simulink model "BswSigProc"  (R2022b)
settings: Solver=FixedStepDiscrete  StartTime=0.0  StopTime=10.0  FixedStep=0.01  RelTol=1e-3
model workspace (MAT data in the model file):
  NvMBlockRecordSignal = Simulink.Signal
  NvMBlockRecordSignal.DataType = 'UInt8'
  NvMBlockRecordSignal.Dimensions = [16 1]
  NvMBlockRecordSignal.DimensionsMode = 'auto'
  NvMBlockRecordSignal.Complexity = 'real'
  NvMBlockRecordSignal.SampleTime = -1
  NvMBlockRecordSignal.SamplingMode = 'auto'
  NvMBlockRecordSignal.InitialValue = 'NvMBlockRecordSignalInit'
  NvMBlockRecordSignalInit = Simulink.Parameter
  NvMBlockRecordSignalInit.Value = [255; 255; 255; 255; 255; 255; 255; 255; 255; 255; 255; 255; 255; 255; 255; 255]
  NvMBlockRecordSignalInit.DataType = 'UInt8'
  NvMBlockRecordSignalInit.Complexity = 'real'
  NvMBlockRecordSignalInit.Dimensions = [16 1]
(part 1 of 2)
settings Model: Creator=blue.ge  LastModifiedBy=blue.ge
settings DataIOCC: SaveFormat=StructureWithTime
settings OptimizationCC: DefaultParameterBehavior=Inlined  EnhancedBackFolding=on  PassReuseOutputArgsAs=Individual arguments  ZeroExternalMemoryAtStartup=off  ZeroInternalMemoryAtStartup=off  OptimizeBlockOrder=speed  DifferentSizesBufferReuse=on  OptimizationCustomize=off  EfficientTunableParamExpr=on
settings DebuggingCC: ParameterTunabilityLossMsg=error
settings ModelReferenceCC: ModelReferenceNumInstancesAllowed=Single
settings SFSimCC: MATLABDynamicMemAlloc=off
settings RTWCC: Description=AUTOSAR  SystemTargetFile=autosar.tlc  GenCodeOnly=on  TemplateMakefile=ert_default_tmf
settings CodeAppCC: ForceParamTrailComments=on  IgnoreCustomStorageClasses=off  OperatorAnnotations=on  SimulinkDataObjDesc=on  SFDataObjDesc=on  InsertBlockDesc=on
settings STFCustomTargetCC: TargetLangStandard=C99 (ISO)  MultiwordLength=256  ParMdlRefBuildCompliant=on  CompOptLevelCompliant=on  IncludeMdlTerminateFcn=off  CombineOutputUpdateFcns=on  SuppressErrorStatus=on  ERTCustomFileBanners=on  SupportNonFinite=off  SupportComplex=off  SupportContinuousTime=off  SupportNonInlinedSFcns=off  RemoveResetFunc=on  MATLABClassNameForMDSCustomization=Simulink.SoftwareTarget.ERTCustomization  AutosarCompliant=on  UseToolchainInfoCompliant=on  LookupTableObjectStructAxisOrder=2,1,3,4,...  LUTObjectStructOrderEvenSpacing=Size,Table,Breakpoints  SystemTargetFile=autosar.tlc
settings ERTTargetCC: TargetLangStandard=C99 (ISO)  ConcurrentExecutionCompliant=off  IncludeMdlTerminateFcn=off  SuppressErrorStatus=on  SupportNonFinite=off  SupportComplex=off  ModelStepFunctionPrototypeControlCompliant=off  CPPClassGenCompliant=off  AutosarCompliant=on  LookupTableObjectStructAxisOrder=2,1,3,4,...  LUTObjectStructOrderEvenSpacing=Size,Table,Breakpoints  InlinedParameterPlacement=NonHierarchical  ERTCustomFileTemplate=example_file_process.tlc

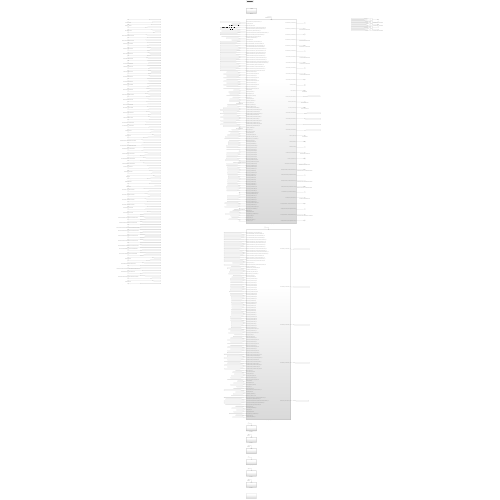
[thumbnail: Simulink's stored preview of the top-level diagram]
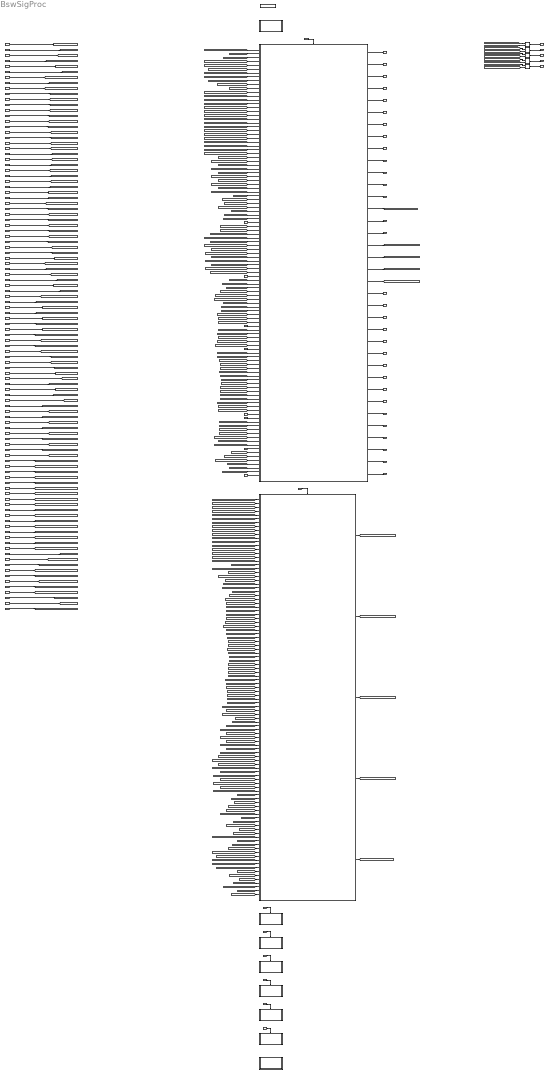
== system BswSigProc ==
  BswSigProc_Runnable : Inport  SampleTime=0.02  OutputFunctionCall=on
  BswSigProc_SignalDeal : Inport  Port=2  SampleTime=0.05  OutputFunctionCall=on
  Xcp_Event0 : Inport  Port=3  SampleTime=0.01  OutputFunctionCall=on
  Xcp_Event1 : Inport  Port=4  SampleTime=0.02  OutputFunctionCall=on
  Xcp_Event2 : Inport  Port=5  SampleTime=0.05  OutputFunctionCall=on
  Xcp_Event3 : Inport  Port=6  SampleTime=0.1  OutputFunctionCall=on
  Xcp_Event4 : Inport  Port=7  SampleTime=0.2  OutputFunctionCall=on
  Xcp_Event5 : Inport  Port=8  SampleTime=1  OutputFunctionCall=on
  ClimaTiMgrVFCInfotainmentPush_ClimaTiMgrVFCInfotainmentPush : Inport  Port=9  OutDataTypeStr=Enum: OnOff1  PortDimensions=1
  ClimRlyCmd_ClimRlyCmd : Inport  Port=10  OutDataTypeStr=Enum: OnOff1  PortDimensions=1
  CmptmtTFrntRaw_CmptmtTFrntRaw : Inport  Port=11  OutDataTypeStr=Bus: CmptmtTFrnt  PortDimensions=1  BusOutputAsStruct=on
  PrkgClimaActvMgrVFCBodyPreClimatisation_PrkgClimaActvMgrVFCBodyPreClimatisation : Inport  Port=12  OutDataTypeStr=Boolean  PortDimensions=1
  PrkgClimaActvMgrVFCGlobalShortDuration_PrkgClimaActvMgrVFCGlobalShortDuration : Inport  Port=13  OutDataTypeStr=Boolean  PortDimensions=1
  PrkgClimaActvMgrVFCLocking_PrkgClimaActvMgrVFCLocking : Inport  Port=14  OutDataTypeStr=Boolean  PortDimensions=1
  PrkgClimaActvMgrVFCParkingDrivingClimatization_PrkgClimaActvMgrVFCParkingDrivingClimatization : Inport  Port=15  OutDataTypeStr=Boolean  PortDimensions=1
  PrkgClimaActvMgrVFCPostClimatisation_PrkgClimaActvMgrVFCPostClimatisation : Inport  Port=16  OutDataTypeStr=Boolean  PortDimensions=1
  PrkgClimaActvMgrVFCWindows_PrkgClimaActvMgrVFCWindows : Inport  Port=17  OutDataTypeStr=Boolean  PortDimensions=1
  PwrGernalOn2OffCnt_PwrMdGernalOn2OffCnt : Inport  Port=18  OutDataTypeStr=On2OffCnt  PortDimensions=1
  RlyCmdCmft_RlyCmdCmft : Inport  Port=19  OutDataTypeStr=Enum: OnOff1  PortDimensions=1
  ActrPosnFrntActForOsaAndRecirc_ActrPosnFrntActForOsaAndRecirc : Inport  Port=20  OutMin=0  OutMax=100  OutDataTypeStr=Perc9  PortDimensions=1
  ActrPosnFrntActForRowFirstModDistbn_ActrPosnFrntActForRowFirstModDistbn : Inport  Port=21  OutMin=0  OutMax=100  OutDataTypeStr=Perc9  PortDimensions=1
  ActrPosnFrntActForRowSecModDistbnLe_ActrPosnFrntActForRowSecModDistbnLe : Inport  Port=22  OutMin=0  OutMax=100  OutDataTypeStr=Perc9  PortDimensions=1
  ActrPosnFrntActForRowSecModDistbnRi_ActrPosnFrntActForRowSecModDistbnRi : Inport  Port=23  OutMin=0  OutMax=100  OutDataTypeStr=Perc9  PortDimensions=1
  AirFlapPosnFrntActForRowFirstForActrDefrst_AirFlapPosnFrntActForRowFirstForActrDefrst : Inport  Port=24  OutMin=0  OutMax=100  OutDataTypeStr=Perc9  PortDimensions=1
  AirFlapPosnFrntActForRowFirstForActrFlrLe_AirFlapPosnFrntActForRowFirstForActrFlrLe : Inport  Port=25  OutMin=0  OutMax=100  OutDataTypeStr=Perc9  PortDimensions=1
  AirFlapPosnFrntActForRowFirstForActrFlrRi_AirFlapPosnFrntActForRowFirstForActrFlrRi : Inport  Port=26  OutMin=0  OutMax=100  OutDataTypeStr=Perc9  PortDimensions=1
  AirFlapPosnFrntActForRowFirstForActrTLe_AirFlapPosnFrntActForRowFirstForActrTLe : Inport  Port=27  OutMin=0  OutMax=100  OutDataTypeStr=Perc9  PortDimensions=1
  AirFlapPosnFrntActForRowFirstForActrTRi_AirFlapPosnFrntActForRowFirstForActrTRi : Inport  Port=28  OutMin=0  OutMax=100  OutDataTypeStr=Perc9  PortDimensions=1
  AirFlapPosnFrntActForRowFirstForActrVentLe_AirFlapPosnFrntActForRowFirstForActrVentLe : Inport  Port=29  OutMin=0  OutMax=100  OutDataTypeStr=Perc9  PortDimensions=1
  AirFlapPosnFrntActForRowFirstForActrVentRi_AirFlapPosnFrntActForRowFirstForActrVentRi : Inport  Port=30  OutMin=0  OutMax=100  OutDataTypeStr=Perc9  PortDimensions=1
  AirFlapPosnFrntActForRowFirstForActrVentSideLe_AirFlapPosnFrntActForRowFirstForActrVentSideLe : Inport  Port=31  OutMin=0  OutMax=100  OutDataTypeStr=Perc9  PortDimensions=1
  AirFlapPosnFrntActForRowFirstForActrVentSideRi_AirFlapPosnFrntActForRowFirstForActrVentSideRi : Inport  Port=32  OutMin=0  OutMax=100  OutDataTypeStr=Perc9  PortDimensions=1
  AirFlapPosnFrntActForRowFirstForOsa_AirFlapPosnFrntActForRowFirstForOsa : Inport  Port=33  OutMin=0  OutMax=100  OutDataTypeStr=Perc9  PortDimensions=1
  AirFlapPosnFrntActForRowFirstForRecirc_AirFlapPosnFrntActForRowFirstForRecirc : Inport  Port=34  OutMin=0  OutMax=100  OutDataTypeStr=Perc9  PortDimensions=1
  AirFlapPosnFrntActForRowSecForActrTLe_AirFlapPosnFrntActForRowSecForActrTLe : Inport  Port=35  OutMin=0  OutMax=100  OutDataTypeStr=Perc9  PortDimensions=1
  AirFlapPosnFrntActForRowSecForActrTRi_AirFlapPosnFrntActForRowSecForActrTRi : Inport  Port=36  OutMin=0  OutMax=100  OutDataTypeStr=Perc9  PortDimensions=1
  AirFragCh1RunngSts_AirFragCh1RunngSts : Inport  Port=37  OutDataTypeStr=Enum: OnOff1  PortDimensions=1
  AirFragCh1RunngSts_AirFragCh1RunngSts_IsUpdated : Inport  Port=38  OutDataTypeStr=boolean  PortDimensions=1
  AirFragCh2RunngSts_AirFragCh2RunngSts : Inport  Port=39  OutDataTypeStr=Enum: OnOff1  PortDimensions=1
  AirFragCh2RunngSts_AirFragCh2RunngSts_IsUpdated : Inport  Port=40  OutDataTypeStr=boolean  PortDimensions=1
  AirFragCh3RunngSts_AirFragCh3RunngSts : Inport  Port=41  OutDataTypeStr=Enum: OnOff1  PortDimensions=1
  AirFragCh3RunngSts_AirFragCh3RunngSts_IsUpdated : Inport  Port=42  OutDataTypeStr=boolean  PortDimensions=1
  AirFragCh4RunngSts_AirFragCh4RunngSts : Inport  Port=43  OutDataTypeStr=Enum: OnOff1  PortDimensions=1
  AirFragCh4RunngSts_AirFragCh4RunngSts_IsUpdated : Inport  Port=44  OutDataTypeStr=boolean  PortDimensions=1
  AirFragCh5RunngSts_AirFragCh5RunngSts : Inport  Port=45  OutDataTypeStr=Enum: OnOff1  PortDimensions=1
  AirFragCh5RunngSts_AirFragCh5RunngSts_IsUpdated : Inport  Port=46  OutDataTypeStr=boolean  PortDimensions=1
  AmbTRaw_AmbTRaw : Inport  Port=47  OutDataTypeStr=Bus: AmbTWithQly  PortDimensions=1  BusOutputAsStruct=on
  BatteryVolValue_BatteryVolValue : Inport  Port=48  OutDataTypeStr=BatteryVolValue  PortDimensions=1
  BkpOfDstTrvld_BkpOfDstTrvld : Inport  Port=49  OutDataTypeStr=Len31  PortDimensions=1
  BkpOfDstTrvld_BkpOfDstTrvld_IsUpdated : Inport  Port=50  OutDataTypeStr=boolean  PortDimensions=1
  CarTiGlb_CarTiGlb : Inport  Port=51  OutDataTypeStr=CarTiGlb  PortDimensions=1
  CarTiGlb_CarTiGlb_IsUpdated : Inport  Port=52  OutDataTypeStr=boolean  PortDimensions=1
  CmptmtTRearRaw_CmptmtTRearRaw : Inport  Port=53  OutDataTypeStr=Bus: CmptmtTFrnt  PortDimensions=1  BusOutputAsStruct=on
  EvaprTReRaw_EvaprTReRaw : Inport  Port=54  OutDataTypeStr=Bus: EvaprTFrnt  PortDimensions=1  BusOutputAsStruct=on
  FrntHvacBlowerSts_FrntHvacBlowerSts : Inport  Port=55  OutDataTypeStr=Enum: HvacFanSts  PortDimensions=1
  HmiHvacFanLvlFrnt_HmiHvacFanLvlFrnt : Inport  Port=56  OutDataTypeStr=Enum: HmiHvacFanLvl  PortDimensions=1
  HvacFanSts2HvacFanBattI2_HvacFanSts2HvacFanBattI2 : Inport  Port=57  OutMin=0  OutMax=63.25  OutDataTypeStr=BlowerReI  PortDimensions=1
  HvacFanSts2HvacFanBattI2_HvacFanSts2HvacFanBattI2_IsUpdated : Inport  Port=58  OutDataTypeStr=boolean  PortDimensions=1
  HvacFanSts2HvacFanBattU2_HvacFanSts2HvacFanBattU2 : Inport  Port=59  OutMin=0  OutMax=25.400000000000002  OutDataTypeStr=U8  PortDimensions=1
  HvacFanSts2HvacFanBattU2_HvacFanSts2HvacFanBattU2_IsUpdated : Inport  Port=60  OutDataTypeStr=boolean  PortDimensions=1
  HvacFanSts3HvacFanBattI_HvacFanSts3HvacFanBattI : Inport  Port=61  OutDataTypeStr=BlowerBattI  PortDimensions=1
  HvacFanSts3HvacFanBattI_HvacFanSts3HvacFanBattI_IsUpdated : Inport  Port=62  OutDataTypeStr=boolean  PortDimensions=1
  HvacFanSts3HvacFanBattU_HvacFanSts3HvacFanBattU : Inport  Port=63  OutDataTypeStr=U10  PortDimensions=1
  HvacFanSts3HvacFanBattU_HvacFanSts3HvacFanBattU_IsUpdated : Inport  Port=64  OutDataTypeStr=boolean  PortDimensions=1
  HvacFanSts3HvacFanSpdFd_HvacFanSts3HvacFanSpdFd : Inport  Port=65  OutDataTypeStr=BlowerSpd  PortDimensions=1
  HvacFanSts3HvacFanSpdFd_HvacFanSts3HvacFanSpdFd_IsUpdated : Inport  Port=66  OutDataTypeStr=boolean  PortDimensions=1
  HvacOsaAndRecActrQfForHp_HvacOsaAndRecActrQfForHp : Inport  Port=67  OutDataTypeStr=Enum: Err1  PortDimensions=1
  HvacReHexAirT_HvacReHexAirT : Inport  Port=68  OutDataTypeStr=Bus: HvacAirTForHeatrFrnt  PortDimensions=1  BusOutputAsStruct=on
  IntCo2Conc_IntCo2Conc : Inport  Port=69  OutDataTypeStr=Co2Conc  PortDimensions=1
  IntCo2Conc_IntCo2Conc_IsUpdated : Inport  Port=70  OutDataTypeStr=boolean  PortDimensions=1
  IntCo2RunSts_IntCo2RunSts : Inport  Port=71  OutDataTypeStr=Enum: PmSnsrSts  PortDimensions=1
  IntCo2RunSts_IntCo2RunSts_IsUpdated : Inport  Port=72  OutDataTypeStr=boolean  PortDimensions=1
  RlyCmftForClimaReq_Ngcc_RlyCmftForClimaReq : Inport  Port=73  OutDataTypeStr=Enum: OnOff1  PortDimensions=1
  RlyCrashForClimaReq_Ngcc_RlyCrashForClimaReq : Inport  Port=74  OutDataTypeStr=Enum: OnOff1  PortDimensions=1
  SysServCarCfgSts_SysServCarID : Inport  Port=75  OutDataTypeStr=Enum: SysServCarID  PortDimensions=1
  SysServCarCfgSts_SysServCCPAQSSts : Inport  Port=76  OutDataTypeStr=UInt8  PortDimensions=1
  SysServCarCfgSts_SysServCCPBGMNum : Inport  Port=77  OutDataTypeStr=UInt8  PortDimensions=1
  SysServCarCfgSts_SysServCCPHEXSensorSts : Inport  Port=78  OutDataTypeStr=UInt8  PortDimensions=1
  SysServCarCfgSts_SysServCCPLin2AFUSts : Inport  Port=79  OutDataTypeStr=Boolean  PortDimensions=1
  SysServCarCfgSts_SysServCCPLin2AIUSts : Inport  Port=80  OutDataTypeStr=UInt8  PortDimensions=1
  SysServCarCfgSts_SysServCCPLin2AQSSts : Inport  Port=81  OutDataTypeStr=Boolean  PortDimensions=1
  SysServCarCfgSts_SysServCCPLin2CDSSts : Inport  Port=82  OutDataTypeStr=Boolean  PortDimensions=1
  SysServCarCfgSts_SysServCCPLin2PMSISts : Inport  Port=83  OutDataTypeStr=Boolean  PortDimensions=1
  SysServCarCfgSts_SysServCCPLin3VGASts : Inport  Port=84  OutDataTypeStr=Boolean  PortDimensions=1
  SysServCarCfgSts_SysServDUAirVentSnrSts : Inport  Port=85  OutDataTypeStr=Boolean  PortDimensions=1
  SysServCarCfgSts_SysServDUBlowerPwmSignSts : Inport  Port=86  OutDataTypeStr=Boolean  PortDimensions=1
  SysServCarCfgSts_SysServDUBlowerPwmSignTypeSts : Inport  Port=87  OutDataTypeStr=UInt8  PortDimensions=1
  SysServCarCfgSts_SysServDUBlowerPwmSts : Inport  Port=88  OutDataTypeStr=Boolean  PortDimensions=1
  SysServCarCfgSts_SysServDUDCDefrostSts : Inport  Port=89  OutDataTypeStr=UInt8  PortDimensions=1
  SysServCarCfgSts_SysServDUDCFLMdeSts : Inport  Port=90  OutDataTypeStr=UInt8  PortDimensions=1
  SysServCarCfgSts_SysServDUDCModeSts : Inport  Port=91  OutDataTypeStr=UInt8  PortDimensions=1
  SysServCarCfgSts_SysServDUDCRecSts : Inport  Port=92  OutDataTypeStr=UInt8  PortDimensions=1
  SysServCarCfgSts_SysServDUDCSModeSts : Inport  Port=93  OutDataTypeStr=UInt8  PortDimensions=1
  SysServCarCfgSts_SysServDUDCSTLSts : Inport  Port=94  OutDataTypeStr=UInt8  PortDimensions=1
  SysServCarCfgSts_SysServDUDCTLSts : Inport  Port=95  OutDataTypeStr=UInt8  PortDimensions=1
  SysServCarCfgSts_SysServDUDCTRSts : Inport  Port=96  OutDataTypeStr=UInt8  PortDimensions=1
  SysServCarCfgSts_SysServDUFLFootSts : Inport  Port=97  OutDataTypeStr=UInt8  PortDimensions=1
  SysServCarCfgSts_SysServDUFLVentSts : Inport  Port=98  OutDataTypeStr=UInt8  PortDimensions=1
  SysServCarCfgSts_SysServDUFRFootSts : Inport  Port=99  OutDataTypeStr=UInt8  PortDimensions=1
  SysServCarCfgSts_SysServDUFRVentSts : Inport  Port=100  OutDataTypeStr=UInt8  PortDimensions=1
  SysServCarCfgSts_SysServDUIncarMotorSts : Inport  Port=101  OutDataTypeStr=Boolean  PortDimensions=1
  SysServCarCfgSts_SysServDULin1HBMFSts : Inport  Port=102  OutDataTypeStr=Boolean  PortDimensions=1
  SysServCarCfgSts_SysServDULin4HFAFSts : Inport  Port=103  OutDataTypeStr=UInt32  PortDimensions=1
  SysServCarCfgSts_SysServDUReBlowerPwmSignSts : Inport  Port=104  OutDataTypeStr=UInt8  PortDimensions=1
  SysServCarCfgSts_SysServDUReBlowerPwmSts : Inport  Port=105  OutDataTypeStr=UInt8  PortDimensions=1
  SysServCarCfgSts_SysServDUSFLFootSts : Inport  Port=106  OutDataTypeStr=UInt8  PortDimensions=1
  SysServCarCfgSts_SysServDUSFLVentSts : Inport  Port=107  OutDataTypeStr=UInt8  PortDimensions=1
  SysServCarCfgSts_SysServDUSFRFootSts : Inport  Port=108  OutDataTypeStr=UInt8  PortDimensions=1
  SysServCarCfgSts_SysServDUSFRVentSts : Inport  Port=109  OutDataTypeStr=UInt8  PortDimensions=1
  SysServCarCfgSts_SysServDUSnsrForFCmptmtTSts : Inport  Port=110  OutDataTypeStr=UInt8  PortDimensions=1
  SysServCarCfgSts_SysServDUSnsrForRair : Inport  Port=111  OutDataTypeStr=UInt8  PortDimensions=1
  SysServCarCfgSts_SysServDUSnsrForRCmptmtTSts : Inport  Port=112  OutDataTypeStr=UInt8  PortDimensions=1
  SysServCarCfgSts_SysServLowSideRETXVSts : Inport  Port=113  OutDataTypeStr=UInt8  PortDimensions=1
  VehBattU_VehBattU : Inport  Port=114  OutDataTypeStr=Bus: SysU  PortDimensions=1  BusOutputAsStruct=on
  VehBattU_VehBattU_IsUpdated : Inport  Port=115  OutDataTypeStr=boolean  PortDimensions=1
  VehModMngtGlbSafe1_Ngcc_VehModMngtGlbSafe1 : Inport  Port=116  OutDataTypeStr=Bus: VehModMngtGlbSafe1  PortDimensions=1  BusOutputAsStruct=on
  VehSpdLgt_Ngcc_VehSpdLgt : Inport  Port=117  OutDataTypeStr=Bus: VehSpdLgtSafe1  PortDimensions=1  BusOutputAsStruct=on
  VFCInfoEna_VFCInfoEna : Inport  Port=118  OutDataTypeStr=Enum: EnableDisableCoding  PortDimensions=1
  VFCInfoEna_VFCInfoEna_IsUpdated : Inport  Port=119  OutDataTypeStr=boolean  PortDimensions=1
  BswLifeCycle_LifeCycle : Inport  Port=120  OutDataTypeStr=Enum: CCMLifeCycleModeGroup  PortDimensions=1
  BswSigProcDtcService : Subsystem
  BswSigProc_Init : Subsystem
  BswSigProc_Runnable_sys : Subsystem
  BswSigProc_SignalDeal_sys : Subsystem
  CcmIntelliClimaResd1_CcmIntelliClimaResd1_merge : Merge
  CcmIntelliClimaResd2_CcmIntelliClimaResd2_merge : Merge
  CcmIntelliClimaResd3_CcmIntelliClimaResd3_merge : Merge
  CcmIntelliClimaResd4_CcmIntelliClimaResd4_merge : Merge
  HvacRecFlapActlPosn_HvacRecFlapActlPosn_merge : Merge
  NvMBlockRecordSignal : DataStoreMemory  DataStoreName=NvMBlockRecordSignal  InitialValue=NvMBlockRecordSignalInit  ShowAdditionalParam=off  Dimensions=16  StateMustResolveToSignalObject=on
  Xcp_Event0_sys : Subsystem
  Xcp_Event1_sys : Subsystem
  Xcp_Event2_sys : Subsystem
  Xcp_Event3_sys : Subsystem
  Xcp_Event4_sys : Subsystem
  Xcp_Event5_sys : Subsystem
  CcmIntelliClimaResd10_CcmIntelliClimaResd10 : Outport  OutDataTypeStr=UInt16  PortDimensions=1
  CcmIntelliClimaResd11_CcmIntelliClimaResd11 : Outport  Port=2  OutDataTypeStr=UInt16  PortDimensions=1
  CcmIntelliClimaResd12_CcmIntelliClimaResd12 : Outport  Port=3  OutDataTypeStr=UInt16  PortDimensions=1
  CcmIntelliClimaResd13_CcmIntelliClimaResd13 : Outport  Port=4  OutDataTypeStr=UInt16  PortDimensions=1
  CcmIntelliClimaResd14_CcmIntelliClimaResd14 : Outport  Port=5  OutDataTypeStr=UInt16  PortDimensions=1
  CcmIntelliClimaResd15_CcmIntelliClimaResd15 : Outport  Port=6  OutDataTypeStr=UInt16  PortDimensions=1
  CcmIntelliClimaResd5_CcmIntelliClimaResd5 : Outport  Port=7  OutDataTypeStr=UInt16  PortDimensions=1
  CcmIntelliClimaResd6_CcmIntelliClimaResd6 : Outport  Port=8  OutDataTypeStr=UInt16  PortDimensions=1
  CcmIntelliClimaResd7_CcmIntelliClimaResd7 : Outport  Port=9  OutDataTypeStr=UInt16  PortDimensions=1
  CcmIntelliClimaResd8_CcmIntelliClimaResd8 : Outport  Port=10  OutDataTypeStr=UInt16  PortDimensions=1
  CcmIntelliClimaResd9_CcmIntelliClimaResd9 : Outport  Port=11  OutDataTypeStr=UInt16  PortDimensions=1
  CmptmtTRe_CmptmtTRe : Outport  Port=12  OutDataTypeStr=Bus: CmptmtTFrnt  PortDimensions=1  BusOutputAsStruct=on
  EvaprTRe_EvaprTRe : Outport  Port=13  OutDataTypeStr=Bus: EvaprTFrnt  PortDimensions=1  BusOutputAsStruct=on
  HvacRecFlapActlPosn_HvacRecFlapActlPosn : Outport  Port=14  OutMin=0  OutMax=100  OutDataTypeStr=Perc9  PortDimensions=1
  ReHvacHexAirT_ReHvacHexAirT : Outport  Port=15  OutDataTypeStr=Bus: HvacAirTForHeatrFrnt  PortDimensions=1  BusOutputAsStruct=on
  VFCVectorCCM_VFCVectorCCM : Outport  Port=16  OutDataTypeStr=Bus: VFCVector  PortDimensions=1  BusOutputAsStruct=on
  CcmIntelliClimaResd1_CcmIntelliClimaResd1 : Outport  Port=17  OutDataTypeStr=UInt8  PortDimensions=1
  CcmIntelliClimaResd2_CcmIntelliClimaResd2 : Outport  Port=18  OutDataTypeStr=UInt8  PortDimensions=1
  CcmIntelliClimaResd3_CcmIntelliClimaResd3 : Outport  Port=19  OutDataTypeStr=UInt8  PortDimensions=1
  CcmIntelliClimaResd4_CcmIntelliClimaResd4 : Outport  Port=20  OutDataTypeStr=UInt8  PortDimensions=1
  CmptmtTFrnt_CmptmtTFrnt : Outport  Port=21  OutDataTypeStr=Bus: CmptmtTFrnt  PortDimensions=1  BusOutputAsStruct=on
  ComMPNCState_PNCState : Outport  Port=22  OutDataTypeStr=Bus: PNCState  PortDimensions=1  BusOutputAsStruct=on
  ComMPNCState_VFCState : Outport  Port=23  OutDataTypeStr=UInt16  PortDimensions=1
  FrntHvacBlowerSts_sdb_FrntHvacBlowerSts : Outport  Port=24  OutDataTypeStr=Enum: HvacFanSts  PortDimensions=1
  HvacCondsorT_sdb_HvacCondsorT : Outport  Port=25  OutDataTypeStr=Bus: EvaprTFrnt  PortDimensions=1  BusOutputAsStruct=on
  HvacDefrstFlapActlPosn_HvacDefrstFlapActlPosn : Outport  Port=26  OutMin=0  OutMax=101  OutDataTypeStr=Perc101  PortDimensions=1
  HvacModFlapActlPosnFrstRowLe_HvacModFlapActlPosnFrstRowLe : Outport  Port=27  OutMin=0  OutMax=101  OutDataTypeStr=Perc101  PortDimensions=1
  HvacModFlapActlPosnFrstRowRi_HvacModFlapActlPosnFrstRowRi : Outport  Port=28  OutMin=0  OutMax=101  OutDataTypeStr=Perc101  PortDimensions=1
  HvacModFlapActlPosnSecRowLe_HvacModFlapActlPosnSecRowLe : Outport  Port=29  OutMin=0  OutMax=101  OutDataTypeStr=Perc101  PortDimensions=1
  HvacModFlapActlPosnSecRowRi_HvacModFlapActlPosnSecRowRi : Outport  Port=30  OutMin=0  OutMax=101  OutDataTypeStr=Perc101  PortDimensions=1
  HvacOsaAndRecActrQfForHp_sdb_HvacOsaAndRecActrQfForHp : Outport  Port=31  OutDataTypeStr=Enum: Err1  PortDimensions=1
  HvacOsaFlapActlPosn_HvacOsaFlapActlPosn : Outport  Port=32  OutMin=0  OutMax=100  OutDataTypeStr=Perc9  PortDimensions=1
  HvacTempFlapActlPosnFrstRowLe_HvacTempFlapActlPosnFrstRowLe : Outport  Port=33  OutMin=0  OutMax=101  OutDataTypeStr=Perc101  PortDimensions=1
  HvacTempFlapActlPosnFrstRowRi_HvacTempFlapActlPosnFrstRowRi : Outport  Port=34  OutMin=0  OutMax=101  OutDataTypeStr=Perc101  PortDimensions=1
  HvacTempFlapActlPosnSecRowLe_HvacTempFlapActlPosnSecRowLe : Outport  Port=35  OutMin=0  OutMax=101  OutDataTypeStr=Perc101  PortDimensions=1
  HvacTempFlapActlPosnSecRowRi_HvacTempFlapActlPosnSecRowRi : Outport  Port=36  OutMin=0  OutMax=101  OutDataTypeStr=Perc101  PortDimensions=1
  wires:
  EvaprTReRaw_EvaprTReRaw/1 -> BswSigProc_Runnable_sys/46(EvaprTReRaw_EvaprTReRaw_read)
  HvacReHexAirT_HvacReHexAirT/1 -> BswSigProc_Runnable_sys/60(HvacReHexAirT_HvacReHexAirT_read)
  SysServCarCfgSts_SysServCCPLin2AQSSts/1 -> BswSigProc_Runnable_sys/73(SysServCarCfgSts_SysServCCPLin2AQSSts_read)
  SysServCarCfgSts_SysServDUBlowerPwmSignTypeSts/1 -> BswSigProc_Runnable_sys/79(SysServCarCfgSts_SysServDUBlowerPwmSignTypeSts_read)
  SysServCarCfgSts_SysServDUReBlowerPwmSignSts/1 -> BswSigProc_Runnable_sys/96(SysServCarCfgSts_SysServDUReBlowerPwmSignSts_read)
  SysServCarCfgSts_SysServDUReBlowerPwmSts/1 -> BswSigProc_Runnable_sys/97(SysServCarCfgSts_SysServDUReBlowerPwmSts_read)
  SysServCarCfgSts_SysServLowSideRETXVSts/1 -> BswSigProc_Runnable_sys/105(SysServCarCfgSts_SysServLowSideRETXVSts_read)
  BswSigProc_Runnable_sys/1(CcmIntelliClimaResd10_CcmIntelliClimaResd10_write) -> CcmIntelliClimaResd10_CcmIntelliClimaResd10/1
  BswSigProc_Runnable_sys/2(CcmIntelliClimaResd11_CcmIntelliClimaResd11_write) -> CcmIntelliClimaResd11_CcmIntelliClimaResd11/1
  BswSigProc_Runnable_sys/3(CcmIntelliClimaResd12_CcmIntelliClimaResd12_write) -> CcmIntelliClimaResd12_CcmIntelliClimaResd12/1
  BswSigProc_Runnable_sys/4(CcmIntelliClimaResd13_CcmIntelliClimaResd13_write) -> CcmIntelliClimaResd13_CcmIntelliClimaResd13/1
  BswSigProc_Runnable_sys/5(CcmIntelliClimaResd14_CcmIntelliClimaResd14_write) -> CcmIntelliClimaResd14_CcmIntelliClimaResd14/1
  BswSigProc_Runnable_sys/6(CcmIntelliClimaResd15_CcmIntelliClimaResd15_write) -> CcmIntelliClimaResd15_CcmIntelliClimaResd15/1
  BswSigProc_Runnable_sys/7(CcmIntelliClimaResd5_CcmIntelliClimaResd5_write) -> CcmIntelliClimaResd5_CcmIntelliClimaResd5/1
  BswSigProc_Runnable_sys/8(CcmIntelliClimaResd6_CcmIntelliClimaResd6_write) -> CcmIntelliClimaResd6_CcmIntelliClimaResd6/1
  BswSigProc_Runnable_sys/9(CcmIntelliClimaResd7_CcmIntelliClimaResd7_write) -> CcmIntelliClimaResd7_CcmIntelliClimaResd7/1
  BswSigProc_Runnable_sys/10(CcmIntelliClimaResd8_CcmIntelliClimaResd8_write) -> CcmIntelliClimaResd8_CcmIntelliClimaResd8/1
  BswSigProc_Runnable_sys/11(CcmIntelliClimaResd9_CcmIntelliClimaResd9_write) -> CcmIntelliClimaResd9_CcmIntelliClimaResd9/1
  BswSigProc_Runnable_sys/12(CmptmtTRe_CmptmtTRe_write) -> CmptmtTRe_CmptmtTRe/1
  BswSigProc_Runnable_sys/13(EvaprTRe_EvaprTRe_write) -> EvaprTRe_EvaprTRe/1
  BswSigProc_Runnable_sys/15(ReHvacHexAirT_ReHvacHexAirT_write) -> ReHvacHexAirT_ReHvacHexAirT/1
  BswSigProc_Runnable_sys/16(VFCVectorCCM_VFCVectorCCM_write) -> VFCVectorCCM_VFCVectorCCM/1
  BswSigProc_Runnable_sys/21(CmptmtTFrnt_CmptmtTFrnt_write) -> CmptmtTFrnt_CmptmtTFrnt/1
  BswSigProc_Runnable_sys/22(ComMPNCState_PNCState_write) -> ComMPNCState_PNCState/1
  BswSigProc_Runnable_sys/23(ComMPNCState_VFCState_write) -> ComMPNCState_VFCState/1
  BswSigProc_Runnable_sys/24(FrntHvacBlowerSts_sdb_FrntHvacBlowerSts_write) -> FrntHvacBlowerSts_sdb_FrntHvacBlowerSts/1
  BswSigProc_Runnable_sys/25(HvacCondsorT_sdb_HvacCondsorT_write) -> HvacCondsorT_sdb_HvacCondsorT/1
  BswSigProc_Runnable_sys/26(HvacDefrstFlapActlPosn_HvacDefrstFlapActlPosn_write) -> HvacDefrstFlapActlPosn_HvacDefrstFlapActlPosn/1
  BswSigProc_Runnable_sys/27(HvacModFlapActlPosnFrstRowLe_HvacModFlapActlPosnFrstRowLe_write) -> HvacModFlapActlPosnFrstRowLe_HvacModFlapActlPosnFrstRowLe/1
  BswSigProc_Runnable_sys/28(HvacModFlapActlPosnFrstRowRi_HvacModFlapActlPosnFrstRowRi_write) -> HvacModFlapActlPosnFrstRowRi_HvacModFlapActlPosnFrstRowRi/1
  BswSigProc_Runnable_sys/29(HvacModFlapActlPosnSecRowLe_HvacModFlapActlPosnSecRowLe_write) -> HvacModFlapActlPosnSecRowLe_HvacModFlapActlPosnSecRowLe/1
  BswSigProc_Runnable_sys/30(HvacModFlapActlPosnSecRowRi_HvacModFlapActlPosnSecRowRi_write) -> HvacModFlapActlPosnSecRowRi_HvacModFlapActlPosnSecRowRi/1
  BswSigProc_Runnable_sys/31(HvacOsaAndRecActrQfForHp_sdb_HvacOsaAndRecActrQfForHp_write) -> HvacOsaAndRecActrQfForHp_sdb_HvacOsaAndRecActrQfForHp/1
  BswSigProc_Runnable_sys/32(HvacOsaFlapActlPosn_HvacOsaFlapActlPosn_write) -> HvacOsaFlapActlPosn_HvacOsaFlapActlPosn/1
  BswSigProc_Runnable_sys/33(HvacTempFlapActlPosnFrstRowLe_HvacTempFlapActlPosnFrstRowLe_write) -> HvacTempFlapActlPosnFrstRowLe_HvacTempFlapActlPosnFrstRowLe/1
  BswSigProc_Runnable_sys/34(HvacTempFlapActlPosnFrstRowRi_HvacTempFlapActlPosnFrstRowRi_write) -> HvacTempFlapActlPosnFrstRowRi_HvacTempFlapActlPosnFrstRowRi/1
  BswSigProc_Runnable_sys/35(HvacTempFlapActlPosnSecRowLe_HvacTempFlapActlPosnSecRowLe_write) -> HvacTempFlapActlPosnSecRowLe_HvacTempFlapActlPosnSecRowLe/1
  BswSigProc_Runnable_sys/36(HvacTempFlapActlPosnSecRowRi_HvacTempFlapActlPosnSecRowRi_write) -> HvacTempFlapActlPosnSecRowRi_HvacTempFlapActlPosnSecRowRi/1
  BswLifeCycle_LifeCycle/1 -> BswSigProc_Runnable_sys/112(BswLifeCycle_LifeCycle_read)
  CcmIntelliClimaResd1_CcmIntelliClimaResd1_merge/1 -> CcmIntelliClimaResd1_CcmIntelliClimaResd1/1
  CcmIntelliClimaResd2_CcmIntelliClimaResd2_merge/1 -> CcmIntelliClimaResd2_CcmIntelliClimaResd2/1
  CcmIntelliClimaResd3_CcmIntelliClimaResd3_merge/1 -> CcmIntelliClimaResd3_CcmIntelliClimaResd3/1
  CcmIntelliClimaResd4_CcmIntelliClimaResd4_merge/1 -> CcmIntelliClimaResd4_CcmIntelliClimaResd4/1
  HvacRecFlapActlPosn_HvacRecFlapActlPosn_merge/1 -> HvacRecFlapActlPosn_HvacRecFlapActlPosn/1
  BswSigProc_SignalDeal_sys/5(HvacRecFlapActlPosn_HvacRecFlapActlPosn_write) -> [HvacRecFlapActlPosn_HvacRecFlapActlPosn_merge_Tag]
  [HvacRecFlapActlPosn_HvacRecFlapActlPosn_merge_Tag] -> HvacRecFlapActlPosn_HvacRecFlapActlPosn_merge/2
  BswSigProc_Runnable_sys/14(HvacRecFlapActlPosn_HvacRecFlapActlPosn_write) -> [HvacRecFlapActlPosn_HvacRecFlapActlPosn_merge_Tag1]
  [HvacRecFlapActlPosn_HvacRecFlapActlPosn_merge_Tag1] -> HvacRecFlapActlPosn_HvacRecFlapActlPosn_merge/1
  BswSigProc_SignalDeal_sys/4(CcmIntelliClimaResd4_CcmIntelliClimaResd4_write) -> [CcmIntelliClimaResd4_CcmIntelliClimaResd4_merge_Tag]
  [CcmIntelliClimaResd4_CcmIntelliClimaResd4_merge_Tag] -> CcmIntelliClimaResd4_CcmIntelliClimaResd4_merge/2
  BswSigProc_Runnable_sys/20(CcmIntelliClimaResd4_CcmIntelliClimaResd4_write) -> [CcmIntelliClimaResd4_CcmIntelliClimaResd4_merge_Tag1]
  [CcmIntelliClimaResd4_CcmIntelliClimaResd4_merge_Tag1] -> CcmIntelliClimaResd4_CcmIntelliClimaResd4_merge/1
  BswSigProc_SignalDeal_sys/3(CcmIntelliClimaResd3_CcmIntelliClimaResd3_write) -> [CcmIntelliClimaResd3_CcmIntelliClimaResd3_merge_Tag]
  [CcmIntelliClimaResd3_CcmIntelliClimaResd3_merge_Tag] -> CcmIntelliClimaResd3_CcmIntelliClimaResd3_merge/2
  BswSigProc_Runnable_sys/19(CcmIntelliClimaResd3_CcmIntelliClimaResd3_write) -> [CcmIntelliClimaResd3_CcmIntelliClimaResd3_merge_Tag1]
  [CcmIntelliClimaResd3_CcmIntelliClimaResd3_merge_Tag1] -> CcmIntelliClimaResd3_CcmIntelliClimaResd3_merge/1
  BswSigProc_SignalDeal_sys/2(CcmIntelliClimaResd2_CcmIntelliClimaResd2_write) -> [CcmIntelliClimaResd2_CcmIntelliClimaResd2_merge_Tag]
  [CcmIntelliClimaResd2_CcmIntelliClimaResd2_merge_Tag] -> CcmIntelliClimaResd2_CcmIntelliClimaResd2_merge/2
  BswSigProc_Runnable_sys/18(CcmIntelliClimaResd2_CcmIntelliClimaResd2_write) -> [CcmIntelliClimaResd2_CcmIntelliClimaResd2_merge_Tag1]
  [CcmIntelliClimaResd2_CcmIntelliClimaResd2_merge_Tag1] -> CcmIntelliClimaResd2_CcmIntelliClimaResd2_merge/1
  BswSigProc_SignalDeal_sys/1(CcmIntelliClimaResd1_CcmIntelliClimaResd1_write) -> [CcmIntelliClimaResd1_CcmIntelliClimaResd1_merge_Tag]
  [CcmIntelliClimaResd1_CcmIntelliClimaResd1_merge_Tag] -> CcmIntelliClimaResd1_CcmIntelliClimaResd1_merge/2
  BswSigProc_Runnable_sys/17(CcmIntelliClimaResd1_CcmIntelliClimaResd1_write) -> [CcmIntelliClimaResd1_CcmIntelliClimaResd1_merge_Tag1]
  [CcmIntelliClimaResd1_CcmIntelliClimaResd1_merge_Tag1] -> CcmIntelliClimaResd1_CcmIntelliClimaResd1_merge/1
  VFCInfoEna_VFCInfoEna_IsUpdated/1 -> [VFCInfoEna_VFCInfoEna_IsUpdated_Tag]
  [VFCInfoEna_VFCInfoEna_IsUpdated_Tag] -> BswSigProc_Runnable_sys/111(VFCInfoEna_VFCInfoEna_IsUpdated_read)
  [VFCInfoEna_VFCInfoEna_IsUpdated_Tag] -> BswSigProc_SignalDeal_sys/104(VFCInfoEna_VFCInfoEna_IsUpdated_read)
  VFCInfoEna_VFCInfoEna/1 -> [VFCInfoEna_VFCInfoEna_Tag]
  [VFCInfoEna_VFCInfoEna_Tag] -> BswSigProc_Runnable_sys/110(VFCInfoEna_VFCInfoEna_read)
  [VFCInfoEna_VFCInfoEna_Tag] -> BswSigProc_SignalDeal_sys/103(VFCInfoEna_VFCInfoEna_read)
  VehSpdLgt_Ngcc_VehSpdLgt/1 -> [VehSpdLgt_Ngcc_VehSpdLgt_Tag]
  [VehSpdLgt_Ngcc_VehSpdLgt_Tag] -> BswSigProc_Runnable_sys/109(VehSpdLgt_Ngcc_VehSpdLgt_read)
  [VehSpdLgt_Ngcc_VehSpdLgt_Tag] -> BswSigProc_SignalDeal_sys/58(VehSpdLgt_Ngcc_VehSpdLgt_read)
  VehModMngtGlbSafe1_Ngcc_VehModMngtGlbSafe1/1 -> [VehModMngtGlbSafe1_Ngcc_VehModMngtGlbSafe1_Tag]
  [VehModMngtGlbSafe1_Ngcc_VehModMngtGlbSafe1_Tag] -> BswSigProc_Runnable_sys/108(VehModMngtGlbSafe1_Ngcc_VehModMngtGlbSafe1_read)
  [VehModMngtGlbSafe1_Ngcc_VehModMngtGlbSafe1_Tag] -> BswSigProc_SignalDeal_sys/102(VehModMngtGlbSafe1_Ngcc_VehModMngtGlbSafe1_read)
  VehBattU_VehBattU_IsUpdated/1 -> [VehBattU_VehBattU_IsUpdated_Tag]
  [VehBattU_VehBattU_IsUpdated_Tag] -> BswSigProc_Runnable_sys/107(VehBattU_VehBattU_IsUpdated_read)
  [VehBattU_VehBattU_IsUpdated_Tag] -> BswSigProc_SignalDeal_sys/101(VehBattU_VehBattU_IsUpdated_read)
  VehBattU_VehBattU/1 -> [VehBattU_VehBattU_Tag]
  [VehBattU_VehBattU_Tag] -> BswSigProc_Runnable_sys/106(VehBattU_VehBattU_read)
  [VehBattU_VehBattU_Tag] -> BswSigProc_SignalDeal_sys/100(VehBattU_VehBattU_read)
  SysServCarCfgSts_SysServDUSnsrForRCmptmtTSts/1 -> [SysServCarCfgSts_SysServDUSnsrForRCmptmtTSts_Tag]
  [SysServCarCfgSts_SysServDUSnsrForRCmptmtTSts_Tag] -> BswSigProc_Runnable_sys/104(SysServCarCfgSts_SysServDUSnsrForRCmptmtTSts_read)
  [SysServCarCfgSts_SysServDUSnsrForRCmptmtTSts_Tag] -> BswSigProc_SignalDeal_sys/57(SysServCarCfgSts_SysServDUSnsrForRCmptmtTSts_read)
  SysServCarCfgSts_SysServDUSnsrForRair/1 -> [SysServCarCfgSts_SysServDUSnsrForRair_Tag]
  [SysServCarCfgSts_SysServDUSnsrForRair_Tag] -> BswSigProc_Runnable_sys/103(SysServCarCfgSts_SysServDUSnsrForRair_read)
  [SysServCarCfgSts_SysServDUSnsrForRair_Tag] -> BswSigProc_SignalDeal_sys/56(SysServCarCfgSts_SysServDUSnsrForRair_read)
  SysServCarCfgSts_SysServDUSnsrForFCmptmtTSts/1 -> [SysServCarCfgSts_SysServDUSnsrForFCmptmtTSts_Tag]
  [SysServCarCfgSts_SysServDUSnsrForFCmptmtTSts_Tag] -> BswSigProc_Runnable_sys/102(SysServCarCfgSts_SysServDUSnsrForFCmptmtTSts_read)
  [SysServCarCfgSts_SysServDUSnsrForFCmptmtTSts_Tag] -> BswSigProc_SignalDeal_sys/55(SysServCarCfgSts_SysServDUSnsrForFCmptmtTSts_read)
  SysServCarCfgSts_SysServDUSFRVentSts/1 -> [SysServCarCfgSts_SysServDUSFRVentSts_Tag]
  [SysServCarCfgSts_SysServDUSFRVentSts_Tag] -> BswSigProc_Runnable_sys/101(SysServCarCfgSts_SysServDUSFRVentSts_read)
  [SysServCarCfgSts_SysServDUSFRVentSts_Tag] -> BswSigProc_SignalDeal_sys/54(SysServCarCfgSts_SysServDUSFRVentSts_read)
  SysServCarCfgSts_SysServDUSFRFootSts/1 -> [SysServCarCfgSts_SysServDUSFRFootSts_Tag]
  [SysServCarCfgSts_SysServDUSFRFootSts_Tag] -> BswSigProc_Runnable_sys/100(SysServCarCfgSts_SysServDUSFRFootSts_read)
  [SysServCarCfgSts_SysServDUSFRFootSts_Tag] -> BswSigProc_SignalDeal_sys/53(SysServCarCfgSts_SysServDUSFRFootSts_read)
  SysServCarCfgSts_SysServDUSFLVentSts/1 -> [SysServCarCfgSts_SysServDUSFLVentSts_Tag]
  [SysServCarCfgSts_SysServDUSFLVentSts_Tag] -> BswSigProc_Runnable_sys/99(SysServCarCfgSts_SysServDUSFLVentSts_read)
  [SysServCarCfgSts_SysServDUSFLVentSts_Tag] -> BswSigProc_SignalDeal_sys/52(SysServCarCfgSts_SysServDUSFLVentSts_read)
  SysServCarCfgSts_SysServDUSFLFootSts/1 -> [SysServCarCfgSts_SysServDUSFLFootSts_Tag]
  [SysServCarCfgSts_SysServDUSFLFootSts_Tag] -> BswSigProc_Runnable_sys/98(SysServCarCfgSts_SysServDUSFLFootSts_read)
  [SysServCarCfgSts_SysServDUSFLFootSts_Tag] -> BswSigProc_SignalDeal_sys/51(SysServCarCfgSts_SysServDUSFLFootSts_read)
  SysServCarCfgSts_SysServDULin4HFAFSts/1 -> [SysServCarCfgSts_SysServDULin4HFAFSts_Tag]
  [SysServCarCfgSts_SysServDULin4HFAFSts_Tag] -> BswSigProc_Runnable_sys/95(SysServCarCfgSts_SysServDULin4HFAFSts_read)
  [SysServCarCfgSts_SysServDULin4HFAFSts_Tag] -> BswSigProc_SignalDeal_sys/50(SysServCarCfgSts_SysServDULin4HFAFSts_read)
  SysServCarCfgSts_SysServDULin1HBMFSts/1 -> [SysServCarCfgSts_SysServDULin1HBMFSts_Tag]
  [SysServCarCfgSts_SysServDULin1HBMFSts_Tag] -> BswSigProc_Runnable_sys/94(SysServCarCfgSts_SysServDULin1HBMFSts_read)
  [SysServCarCfgSts_SysServDULin1HBMFSts_Tag] -> BswSigProc_SignalDeal_sys/49(SysServCarCfgSts_SysServDULin1HBMFSts_read)
  SysServCarCfgSts_SysServDUIncarMotorSts/1 -> [SysServCarCfgSts_SysServDUIncarMotorSts_Tag]